simulink model "VirtualADAPT"  (Simulink 8.9)
settings: Solver=ode45  StartTime=0.0  StopTime=inf  RelTol=1e-3  EnableMultiTasking=on
PostLoadFcn:
  | FaultInjectionGUIHandle = FaultInjectionGUI;
InitFcn:
  | VirtualADAPTDataStructure
undefined symbols (not defined in the file or in its folder): ln
libraries: simulink x363
(part 8 of 26)

== system VirtualADAPT/VirtualADAPTv1/PowerStorage/RelayEY260/Relay ==
  u(1) : Inport
  u(2) : Inport  Port=2
  u(3) : Inport  Port=3
  u(4) : Inport  Port=4
  Adder : Sum  SaturateOnIntegerOverflow=on  InputSameDT=on  OutDataTypeStr=Inherit: Same as first input
  Gain : Gain  SaturateOnIntegerOverflow=on  OutDataTypeStr=Inherit: Same as input  ParamDataTypeStr=Inherit: Same as input
  Mux : Mux
  OffGuard : Fcn  Expr=(!u(4))
  OnGuard : Fcn  Expr=(u(4))
  Product1 : Product  SaturateOnIntegerOverflow=on  RndMeth=Zero  InputSameDT=on  OutDataTypeStr=Inherit: Same as first input
  Product2 : Product  SaturateOnIntegerOverflow=on  RndMeth=Zero  InputSameDT=on  OutDataTypeStr=Inherit: Same as first input
  determineState : Subsystem
  y(1) : Outport
  y(2) : Outport  Port=2
  y(3) : Outport  Port=3
  O : Outport  Port=4
  State : Outport  Port=5
  wires:
  Adder/1 -> Gain/1
  u(4)/1 -> Mux/4
  Mux/1 -> OnGuard/1, OffGuard/1
  OnGuard/1 -> determineState/1(In1)
  OffGuard/1 -> determineState/2(In2)
  u(1)/1 -> Product1/1
  determineState/1(Out1) -> Product1/2, Product2/2, State/1
  Product1/1 -> O/1, y(2)/1, y(3)/1
  Gain/1 -> Product2/1
  Product2/1 -> y(1)/1
  u(2)/1 -> Adder/1
  u(3)/1 -> Adder/2

== system VirtualADAPT/VirtualADAPTv1/PowerStorage/RelayEY260/Sensor ==
  TrueValue : Inport
  MSe : Subsystem  mask{FP=Nominal, FT=0, FM=0}
      mask prompts: FP "Fault Profile:"; FT "Fault Injection Time:"; FM "Fault Magnitude/Slope/Bias/StuckAtValue"
      mask TypeOptions FP: Nominal | Abrupt | Incipient | Bias | StuckAt
      mask Display: image(imread('MSe.png','BackgroundColor',[1 1 1]))
  R : Subsystem  mask{R=1, FP=Nominal, FT=0, FM=0}
      mask prompts: R "Value of R:"; FP "Fault Profile:"; FT "Fault Injection Time:"; FM "Fault Magnitude/Slope"
      mask TypeOptions FP: Nominal | Abrupt | Incipient
      mask Display: image(imread('R.png','BackgroundColor',[1 1 1]))
  TrueValueMod : Subsystem
      mask Display: image(imread('fx.png','BackgroundColor',[1 1 1]))
  Value : Subsystem
      mask Display: image(imread('0.png','BackgroundColor',[1 1 1]))
  SensedValue : Outport
  wires:
  Value/1(y(1)) -> R/1(In)
  R/1(Out) -> Value/1(u(1))
  MSe/1(E) -> Value/2(u(2))
  Value/2(y(2)) -> MSe/1(F)
  TrueValueMod/1(y(1)) -> MSe/2(Se)
  TrueValue/1 -> TrueValueMod/1(u(1))
  Value/3(O) -> SensedValue/1

== system VirtualADAPT/VirtualADAPTv1/PowerStorage/SensorE161/MSe ==
  F : Inport
  Se : Inport  Port=2
  Bias : Step  Time=FT  After=FM  SampleTime=0
  Fault Inject : Subsystem
  Fault Profile : Constant  Value=FP
  Mult2 : Product  SaturateOnIntegerOverflow=on  RndMeth=Zero  InputSameDT=on  OutDataTypeStr=Inherit: Same as first input
  Multiply : Product  SaturateOnIntegerOverflow=on  RndMeth=Zero  InputSameDT=on  OutDataTypeStr=Inherit: Same as first input
  Ramp : simulink/Sources/Ramp  slope=FM  start=FT  X0=0
  Step : Step  Time=FT  Before=1  After=(1 + FM)  SampleTime=0
  StuckAt : Step  Time=FT  Before=1  After=0  SampleTime=0
  StuckAt2 : Step  Time=FT  After=FM  SampleTime=0
  Sum : Sum  SaturateOnIntegerOverflow=on  InputSameDT=on  OutDataTypeStr=Inherit: Same as first input
  Sum2 : Sum  SaturateOnIntegerOverflow=on  InputSameDT=on  OutDataTypeStr=Inherit: Same as first input
  Sum3 : Sum  SaturateOnIntegerOverflow=on  InputSameDT=on  OutDataTypeStr=Inherit: Same as first input
  E : Outport
  wires:
  Fault Inject/1(Out1) -> E/1
  Se/1 -> Fault Inject/1(Nominal), Multiply/1, Sum/1, Sum2/1, Mult2/1
  Fault Profile/1 -> Fault Inject/2(Fault Profile)
  Step/1 -> Multiply/2
  Ramp/1 -> Sum/2
  Multiply/1 -> Fault Inject/3(Step)
  Sum/1 -> Fault Inject/4(Ramp)
  Bias/1 -> Sum2/2
  Sum2/1 -> Fault Inject/5(Bias)
  StuckAt/1 -> Mult2/2
  Mult2/1 -> Sum3/1
  StuckAt2/1 -> Sum3/2
  Sum3/1 -> Fault Inject/6(stuckAt)

== system VirtualADAPT/VirtualADAPTv1/PowerStorage/SensorE161/R ==
  In : Inport
  Bond : Constant  Value=2
  Computing R : Subsystem
  Fault Profile : Constant  Value=FP
  JuncDB : Constant
  Junction Type : Constant  Value=0
  Ramp : simulink/Sources/Ramp  slope=FM  start=FT  X0=R
  Resistor Value : Constant  Value=R
  Step : Step  Time=FT  Before=R  After=(1 + FM)*R  SampleTime=0
  Out : Outport
  R : Outport  Port=2
  wires:
  Computing R/1(Out1) -> Out/1
  In/1 -> Computing R/1(In1)
  Resistor Value/1 -> Computing R/2(RNominal)
  Fault Profile/1 -> Computing R/3(Fault Profile)
  Step/1 -> Computing R/4(Step)
  Ramp/1 -> Computing R/5(Ramp)
  JuncDB/1 -> Computing R/6(JuncDB)
  Bond/1 -> Computing R/7(Bond)
  Junction Type/1 -> Computing R/8(Junction Type)
  Computing R/2(Out2) -> R/1

== system VirtualADAPT/VirtualADAPTv1/PowerStorage/SensorE161/TrueValueMod ==
  u(1) : Inport
  Function : Fcn  Expr=u(1)
  Mux : Mux  Inputs=1
  y(1) : Outport
  wires:
  u(1)/1 -> Mux/1
  Mux/1 -> Function/1
  Function/1 -> y(1)/1

== system VirtualADAPT/VirtualADAPTv1/PowerStorage/SensorE161/Value ==
  u(1) : Inport
  u(2) : Inport  Port=2
  Adder : Sum  Inputs=-  SaturateOnIntegerOverflow=on  InputSameDT=on  OutDataTypeStr=Inherit: Same as first input
  Gain : Gain  Gain=-1  SaturateOnIntegerOverflow=on  OutDataTypeStr=Inherit: Same as input  ParamDataTypeStr=Inherit: Same as input
  y(1) : Outport
  y(2) : Outport  Port=2
  O : Outport  Port=3
  wires:
  Adder/1 -> Gain/1
  Gain/1 -> y(1)/1
  u(1)/1 -> y(2)/1, O/1
  u(2)/1 -> Adder/1

== system VirtualADAPT/VirtualADAPTv1/PowerStorage/SensorE261/MSe ==
  F : Inport
  Se : Inport  Port=2
  Bias : Step  Time=FT  After=FM  SampleTime=0
  Fault Inject : Subsystem
  Fault Profile : Constant  Value=FP
  Mult2 : Product  SaturateOnIntegerOverflow=on  RndMeth=Zero  InputSameDT=on  OutDataTypeStr=Inherit: Same as first input
  Multiply : Product  SaturateOnIntegerOverflow=on  RndMeth=Zero  InputSameDT=on  OutDataTypeStr=Inherit: Same as first input
  Ramp : simulink/Sources/Ramp  slope=FM  start=FT  X0=0
  Step : Step  Time=FT  Before=1  After=(1 + FM)  SampleTime=0
  StuckAt : Step  Time=FT  Before=1  After=0  SampleTime=0
  StuckAt2 : Step  Time=FT  After=FM  SampleTime=0
  Sum : Sum  SaturateOnIntegerOverflow=on  InputSameDT=on  OutDataTypeStr=Inherit: Same as first input
  Sum2 : Sum  SaturateOnIntegerOverflow=on  InputSameDT=on  OutDataTypeStr=Inherit: Same as first input
  Sum3 : Sum  SaturateOnIntegerOverflow=on  InputSameDT=on  OutDataTypeStr=Inherit: Same as first input
  E : Outport
  wires:
  Fault Inject/1(Out1) -> E/1
  Se/1 -> Fault Inject/1(Nominal), Multiply/1, Sum/1, Sum2/1, Mult2/1
  Fault Profile/1 -> Fault Inject/2(Fault Profile)
  Step/1 -> Multiply/2
  Ramp/1 -> Sum/2
  Multiply/1 -> Fault Inject/3(Step)
  Sum/1 -> Fault Inject/4(Ramp)
  Bias/1 -> Sum2/2
  Sum2/1 -> Fault Inject/5(Bias)
  StuckAt/1 -> Mult2/2
  Mult2/1 -> Sum3/1
  StuckAt2/1 -> Sum3/2
  Sum3/1 -> Fault Inject/6(stuckAt)

== system VirtualADAPT/VirtualADAPTv1/PowerStorage/SensorE261/R ==
  In : Inport
  Bond : Constant  Value=2
  Computing R : Subsystem
  Fault Profile : Constant  Value=FP
  JuncDB : Constant
  Junction Type : Constant  Value=0
  Ramp : simulink/Sources/Ramp  slope=FM  start=FT  X0=R
  Resistor Value : Constant  Value=R
  Step : Step  Time=FT  Before=R  After=(1 + FM)*R  SampleTime=0
  Out : Outport
  R : Outport  Port=2
  wires:
  Computing R/1(Out1) -> Out/1
  In/1 -> Computing R/1(In1)
  Resistor Value/1 -> Computing R/2(RNominal)
  Fault Profile/1 -> Computing R/3(Fault Profile)
  Step/1 -> Computing R/4(Step)
  Ramp/1 -> Computing R/5(Ramp)
  JuncDB/1 -> Computing R/6(JuncDB)
  Bond/1 -> Computing R/7(Bond)
  Junction Type/1 -> Computing R/8(Junction Type)
  Computing R/2(Out2) -> R/1

== system VirtualADAPT/VirtualADAPTv1/PowerStorage/SensorE261/TrueValueMod ==
  u(1) : Inport
  Function : Fcn  Expr=u(1)
  Mux : Mux  Inputs=1
  y(1) : Outport
  wires:
  u(1)/1 -> Mux/1
  Mux/1 -> Function/1
  Function/1 -> y(1)/1

== system VirtualADAPT/VirtualADAPTv1/PowerStorage/SensorE261/Value ==
  u(1) : Inport
  u(2) : Inport  Port=2
  Adder : Sum  Inputs=-  SaturateOnIntegerOverflow=on  InputSameDT=on  OutDataTypeStr=Inherit: Same as first input
  Gain : Gain  Gain=-1  SaturateOnIntegerOverflow=on  OutDataTypeStr=Inherit: Same as input  ParamDataTypeStr=Inherit: Same as input
  y(1) : Outport
  y(2) : Outport  Port=2
  O : Outport  Port=3
  wires:
  Adder/1 -> Gain/1
  Gain/1 -> y(1)/1
  u(1)/1 -> y(2)/1, O/1
  u(2)/1 -> Adder/1

== system VirtualADAPT/VirtualADAPTv1/PowerStorage/SensorIT161/MSe ==
  F : Inport
  Se : Inport  Port=2
  Bias : Step  Time=FT  After=FM  SampleTime=0
  Fault Inject : Subsystem
  Fault Profile : Constant  Value=FP
  Mult2 : Product  SaturateOnIntegerOverflow=on  RndMeth=Zero  InputSameDT=on  OutDataTypeStr=Inherit: Same as first input
  Multiply : Product  SaturateOnIntegerOverflow=on  RndMeth=Zero  InputSameDT=on  OutDataTypeStr=Inherit: Same as first input
  Ramp : simulink/Sources/Ramp  slope=FM  start=FT  X0=0
  Step : Step  Time=FT  Before=1  After=(1 + FM)  SampleTime=0
  StuckAt : Step  Time=FT  Before=1  After=0  SampleTime=0
  StuckAt2 : Step  Time=FT  After=FM  SampleTime=0
  Sum : Sum  SaturateOnIntegerOverflow=on  InputSameDT=on  OutDataTypeStr=Inherit: Same as first input
  Sum2 : Sum  SaturateOnIntegerOverflow=on  InputSameDT=on  OutDataTypeStr=Inherit: Same as first input
  Sum3 : Sum  SaturateOnIntegerOverflow=on  InputSameDT=on  OutDataTypeStr=Inherit: Same as first input
  E : Outport
  wires:
  Fault Inject/1(Out1) -> E/1
  Se/1 -> Fault Inject/1(Nominal), Multiply/1, Sum/1, Sum2/1, Mult2/1
  Fault Profile/1 -> Fault Inject/2(Fault Profile)
  Step/1 -> Multiply/2
  Ramp/1 -> Sum/2
  Multiply/1 -> Fault Inject/3(Step)
  Sum/1 -> Fault Inject/4(Ramp)
  Bias/1 -> Sum2/2
  Sum2/1 -> Fault Inject/5(Bias)
  StuckAt/1 -> Mult2/2
  Mult2/1 -> Sum3/1
  StuckAt2/1 -> Sum3/2
  Sum3/1 -> Fault Inject/6(stuckAt)

== system VirtualADAPT/VirtualADAPTv1/PowerStorage/SensorIT161/R ==
  In : Inport
  Bond : Constant  Value=2
  Computing R : Subsystem
  Fault Profile : Constant  Value=FP
  JuncDB : Constant
  Junction Type : Constant  Value=0
  Ramp : simulink/Sources/Ramp  slope=FM  start=FT  X0=R
  Resistor Value : Constant  Value=R
  Step : Step  Time=FT  Before=R  After=(1 + FM)*R  SampleTime=0
  Out : Outport
  R : Outport  Port=2
  wires:
  Computing R/1(Out1) -> Out/1
  In/1 -> Computing R/1(In1)
  Resistor Value/1 -> Computing R/2(RNominal)
  Fault Profile/1 -> Computing R/3(Fault Profile)
  Step/1 -> Computing R/4(Step)
  Ramp/1 -> Computing R/5(Ramp)
  JuncDB/1 -> Computing R/6(JuncDB)
  Bond/1 -> Computing R/7(Bond)
  Junction Type/1 -> Computing R/8(Junction Type)
  Computing R/2(Out2) -> R/1

== system VirtualADAPT/VirtualADAPTv1/PowerStorage/SensorIT161/TrueValueMod ==
  u(1) : Inport
  Function : Fcn  Expr=u(1)
  Mux : Mux  Inputs=1
  y(1) : Outport
  wires:
  u(1)/1 -> Mux/1
  Mux/1 -> Function/1
  Function/1 -> y(1)/1

== system VirtualADAPT/VirtualADAPTv1/PowerStorage/SensorIT161/Value ==
  u(1) : Inport
  u(2) : Inport  Port=2
  Adder : Sum  Inputs=-  SaturateOnIntegerOverflow=on  InputSameDT=on  OutDataTypeStr=Inherit: Same as first input
  Gain : Gain  Gain=-1  SaturateOnIntegerOverflow=on  OutDataTypeStr=Inherit: Same as input  ParamDataTypeStr=Inherit: Same as input
  y(1) : Outport
  y(2) : Outport  Port=2
  O : Outport  Port=3
  wires:
  Adder/1 -> Gain/1
  Gain/1 -> y(1)/1
  u(1)/1 -> y(2)/1, O/1
  u(2)/1 -> Adder/1

== system VirtualADAPT/VirtualADAPTv1/PowerStorage/SensorIT261/MSe ==
  F : Inport
  Se : Inport  Port=2
  Bias : Step  Time=FT  After=FM  SampleTime=0
  Fault Inject : Subsystem
  Fault Profile : Constant  Value=FP
  Mult2 : Product  SaturateOnIntegerOverflow=on  RndMeth=Zero  InputSameDT=on  OutDataTypeStr=Inherit: Same as first input
  Multiply : Product  SaturateOnIntegerOverflow=on  RndMeth=Zero  InputSameDT=on  OutDataTypeStr=Inherit: Same as first input
  Ramp : simulink/Sources/Ramp  slope=FM  start=FT  X0=0
  Step : Step  Time=FT  Before=1  After=(1 + FM)  SampleTime=0
  StuckAt : Step  Time=FT  Before=1  After=0  SampleTime=0
  StuckAt2 : Step  Time=FT  After=FM  SampleTime=0
  Sum : Sum  SaturateOnIntegerOverflow=on  InputSameDT=on  OutDataTypeStr=Inherit: Same as first input
  Sum2 : Sum  SaturateOnIntegerOverflow=on  InputSameDT=on  OutDataTypeStr=Inherit: Same as first input
  Sum3 : Sum  SaturateOnIntegerOverflow=on  InputSameDT=on  OutDataTypeStr=Inherit: Same as first input
  E : Outport
  wires:
  Fault Inject/1(Out1) -> E/1
  Se/1 -> Fault Inject/1(Nominal), Multiply/1, Sum/1, Sum2/1, Mult2/1
  Fault Profile/1 -> Fault Inject/2(Fault Profile)
  Step/1 -> Multiply/2
  Ramp/1 -> Sum/2
  Multiply/1 -> Fault Inject/3(Step)
  Sum/1 -> Fault Inject/4(Ramp)
  Bias/1 -> Sum2/2
  Sum2/1 -> Fault Inject/5(Bias)
  StuckAt/1 -> Mult2/2
  Mult2/1 -> Sum3/1
  StuckAt2/1 -> Sum3/2
  Sum3/1 -> Fault Inject/6(stuckAt)

== system VirtualADAPT/VirtualADAPTv1/PowerStorage/SensorIT261/R ==
  In : Inport
  Bond : Constant  Value=2
  Computing R : Subsystem
  Fault Profile : Constant  Value=FP
  JuncDB : Constant
  Junction Type : Constant  Value=0
  Ramp : simulink/Sources/Ramp  slope=FM  start=FT  X0=R
  Resistor Value : Constant  Value=R
  Step : Step  Time=FT  Before=R  After=(1 + FM)*R  SampleTime=0
  Out : Outport
  R : Outport  Port=2
  wires:
  Computing R/1(Out1) -> Out/1
  In/1 -> Computing R/1(In1)
  Resistor Value/1 -> Computing R/2(RNominal)
  Fault Profile/1 -> Computing R/3(Fault Profile)
  Step/1 -> Computing R/4(Step)
  Ramp/1 -> Computing R/5(Ramp)
  JuncDB/1 -> Computing R/6(JuncDB)
  Bond/1 -> Computing R/7(Bond)
  Junction Type/1 -> Computing R/8(Junction Type)
  Computing R/2(Out2) -> R/1

== system VirtualADAPT/VirtualADAPTv1/PowerStorage/SensorIT261/TrueValueMod ==
  u(1) : Inport
  Function : Fcn  Expr=u(1)
  Mux : Mux  Inputs=1
  y(1) : Outport
  wires:
  u(1)/1 -> Mux/1
  Mux/1 -> Function/1
  Function/1 -> y(1)/1

== system VirtualADAPT/VirtualADAPTv1/PowerStorage/SensorIT261/Value ==
  u(1) : Inport
  u(2) : Inport  Port=2
  Adder : Sum  Inputs=-  SaturateOnIntegerOverflow=on  InputSameDT=on  OutDataTypeStr=Inherit: Same as first input
  Gain : Gain  Gain=-1  SaturateOnIntegerOverflow=on  OutDataTypeStr=Inherit: Same as input  ParamDataTypeStr=Inherit: Same as input
  y(1) : Outport
  y(2) : Outport  Port=2
  O : Outport  Port=3
  wires:
  Adder/1 -> Gain/1
  Gain/1 -> y(1)/1
  u(1)/1 -> y(2)/1, O/1
  u(2)/1 -> Adder/1

== system VirtualADAPT/VirtualADAPTv1/SensorTE133/MSe/Fault Inject ==
  Nominal : Inport
  Fault Profile : Inport  Port=2
  Step : Inport  Port=3
  Ramp : Inport  Port=4
  Bias : Inport  Port=5
  stuckAt : Inport  Port=6
  Switch1 : MultiPortSwitch  Inputs=5  SaturateOnIntegerOverflow=on  InputSameDT=on
  Out1 : Outport
  wires:
  Nominal/1 -> Switch1/2
  Fault Profile/1 -> Switch1/1
  Step/1 -> Switch1/3
  Ramp/1 -> Switch1/4
  Bias/1 -> Switch1/5
  stuckAt/1 -> Switch1/6
  Switch1/1 -> Out1/1

== system VirtualADAPT/VirtualADAPTv1/SensorTE133/R/Computing R ==
  In1 : Inport
  RNominal : Inport  Port=2
  Fault Profile : Inport  Port=3
  Step : Inport  Port=4
  Ramp : Inport  Port=5
  JuncDB : Inport  Port=6
  Bond : Inport  Port=7
  Junction Type : Inport  Port=8
  CalcCausality : Fcn  Expr=(u(1) == u(2) && u(3) ==1) || (u(1) !=u(2) && u(3) == 0)
  CalcOutput : Fcn  Expr=(u(1)*u(2)^u(3))
  MinusOne : Constant  Value=-1
  Mux1 : Mux  Inputs=3
  Mux2 : Mux  Inputs=3
  One : Constant
  Switch1 : MultiPortSwitch  SaturateOnIntegerOverflow=on  InputSameDT=on
  Switch2 : MultiPortSwitch  DataPortOrder=Zero-based contiguous  Inputs=2  zeroidx=on  SaturateOnIntegerOverflow=on  InputSameDT=on
  Out1 : Outport
  Out2 : Outport  Port=2
  wires:
  In1/1 -> Mux2/1
  Fault Profile/1 -> Switch1/1
  RNominal/1 -> Switch1/2
  Step/1 -> Switch1/3
  Ramp/1 -> Switch1/4
  Switch1/1 -> Out2/1, Mux2/2
  JuncDB/1 -> Mux1/1
  Bond/1 -> Mux1/2
  Junction Type/1 -> Mux1/3
  Mux1/1 -> CalcCausality/1
  CalcCausality/1 -> Switch2/1
  One/1 -> Switch2/2
  MinusOne/1 -> Switch2/3
  Switch2/1 -> Mux2/3
  Mux2/1 -> CalcOutput/1
  CalcOutput/1 -> Out1/1

== system VirtualADAPT/VirtualADAPTv1/SensorTE133/TempBias/Fault Inject ==
  Nominal : Inport
  Fault Profile : Inport  Port=2
  Step : Inport  Port=3
  Ramp : Inport  Port=4
  Bias : Inport  Port=5
  stuckAt : Inport  Port=6
  Switch1 : MultiPortSwitch  Inputs=5  SaturateOnIntegerOverflow=on  InputSameDT=on
  Out1 : Outport
  wires:
  Nominal/1 -> Switch1/2
  Fault Profile/1 -> Switch1/1
  Step/1 -> Switch1/3
  Ramp/1 -> Switch1/4
  Bias/1 -> Switch1/5
  stuckAt/1 -> Switch1/6
  Switch1/1 -> Out1/1

== system VirtualADAPT/VirtualADAPTv1/PowerDistribution/ACLoadBank1/L1A/LGT6 ==
  VInIn0 : Inport
  AmbientTemp : Inport  Port=2
  ComputeCurrent : Subsystem
      mask Display: image(imread('fx.png','BackgroundColor',[1 1 1]))
  ComputePower : Subsystem
      mask Display: image(imread('fx.png','BackgroundColor',[1 1 1]))
  LightBulb1 : Subsystem
  LightBulb2 : Subsystem
  LightBulb3 : Subsystem
  O1 : Subsystem
      mask Display: image(imread('1.png','BackgroundColor',[1 1 1]))
  Z1 : Subsystem
      mask Display: image(imread('0.png','BackgroundColor',[1 1 1]))
  VInOut0 : Outport
  Power : Outport  Port=2
  Temperature1 : Outport  Port=3
  Temperature3 : Outport  Port=4
  Current : Outport  Port=5
  Temperature2 : Outport  Port=6
  wires:
  O1/1(y(1)) -> Z1/1(u(1))
  Z1/1(y(1)) -> O1/1(u(1))
  VInIn0/1 -> O1/2(u(2))
  O1/2(y(2)) -> VInOut0/1
  LightBulb1/1(EPOut0) -> Z1/2(u(2))
  Z1/2(y(2)) -> LightBulb1/1(EPIn0)
  LightBulb2/1(EPOut0) -> Z1/3(u(3))
  Z1/3(y(3)) -> LightBulb2/1(EPIn0)
  LightBulb3/1(EPOut0) -> Z1/4(u(4))
  Z1/4(y(4)) -> LightBulb3/1(EPIn0)
  LightBulb1/4(Power) -> ComputePower/1(u(1))
  LightBulb2/4(Power) -> ComputePower/2(u(2))
  LightBulb3/2(Power) -> ComputePower/3(u(3))
  LightBulb1/2(Current) -> ComputeCurrent/1(u(1))
  LightBulb2/3(Current) -> ComputeCurrent/2(u(2))
  LightBulb3/3(Current) -> ComputeCurrent/3(u(3))
  ComputePower/1(y(1)) -> Power/1
  LightBulb1/3(Temperature) -> Temperature1/1
  LightBulb2/2(Temperature) -> Temperature2/1
  LightBulb3/4(Temperature) -> Temperature3/1
  AmbientTemp/1 -> LightBulb1/2(AmbientTemp), LightBulb2/2(AmbientTemp), LightBulb3/2(AmbientTemp)
  ComputeCurrent/1(y(1)) -> Current/1

== system VirtualADAPT/VirtualADAPTv1/PowerDistribution/ACLoadBank1/L1A/SensorLT500 ==
  Power : Inport
  Bias : Subsystem  mask{Constant=15.411, FP=Nominal, FT=0, FM=0}
      mask prompts: Constant "Value of Constant:"; FP "Fault Profile:"; FT "Fault Injection Time:"; FM "Fault Magnitude/Slope"
      mask TypeOptions FP: Nominal | Abrupt | Incipient
      mask Display: image(imread('Constant.png','BackgroundColor',[1 1 1]))
  ComputeLuminosity : Subsystem
      mask Display: image(imread('fx.png','BackgroundColor',[1 1 1]))
  Gain : Subsystem  mask{Constant=0.56045, FP=Nominal, FT=0, FM=0}
      mask prompts: Constant "Value of Constant:"; FP "Fault Profile:"; FT "Fault Injection Time:"; FM "Fault Magnitude/Slope"
      mask TypeOptions FP: Nominal | Abrupt | Incipient
      mask Display: image(imread('Constant.png','BackgroundColor',[1 1 1]))
  MSe : Subsystem  mask{FP=Nominal, FT=0, FM=0}
      mask prompts: FP "Fault Profile:"; FT "Fault Injection Time:"; FM "Fault Magnitude/Slope/Bias/StuckAtValue"
      mask TypeOptions FP: Nominal | Abrupt | Incipient | Bias | StuckAt
      mask Display: image(imread('MSe.png','BackgroundColor',[1 1 1]))
  R : Subsystem  mask{R=100, FP=Nominal, FT=0, FM=0}
      mask prompts: R "Value of R:"; FP "Fault Profile:"; FT "Fault Injection Time:"; FM "Fault Magnitude/Slope"
      mask TypeOptions FP: Nominal | Abrupt | Incipient
      mask Display: image(imread('R.png','BackgroundColor',[1 1 1]))
  ZeroJunction : Subsystem
      mask Display: image(imread('0.png','BackgroundColor',[1 1 1]))
  Light : Outport
  wires:
  ZeroJunction/1(y(1)) -> R/1(In)
  R/1(Out) -> ZeroJunction/1(u(1))
  MSe/1(E) -> ZeroJunction/2(u(2))
  ZeroJunction/2(y(2)) -> MSe/1(F)
  ComputeLuminosity/1(y(1)) -> MSe/2(Se)
  Bias/1(C) -> ComputeLuminosity/1(u(1))
  Power/1 -> ComputeLuminosity/2(u(2))
  Gain/1(C) -> ComputeLuminosity/3(u(3))
  ZeroJunction/3(O) -> Light/1

== system VirtualADAPT/VirtualADAPTv1/PowerDistribution/ACLoadBank1/L1A/SensorTE500 ==
  TrueValue : Inport
  MSe : Subsystem  mask{FP=Nominal, FT=0, FM=0}
      mask prompts: FP "Fault Profile:"; FT "Fault Injection Time:"; FM "Fault Magnitude/Slope/Bias/StuckAtValue"
      mask TypeOptions FP: Nominal | Abrupt | Incipient | Bias | StuckAt
      mask Display: image(imread('MSe.png','BackgroundColor',[1 1 1]))
  R : Subsystem  mask{R=1, FP=Nominal, FT=0, FM=0}
      mask prompts: R "Value of R:"; FP "Fault Profile:"; FT "Fault Injection Time:"; FM "Fault Magnitude/Slope"
      mask TypeOptions FP: Nominal | Abrupt | Incipient
      mask Display: image(imread('R.png','BackgroundColor',[1 1 1]))
  TempBias : Subsystem  mask{Constant=-1.5, FP=Nominal, FT=0, FM=0}
      mask prompts: Constant "Value of Constant:"; FP "Fault Profile:"; FT "Fault Injection Time:"; FM "Fault Magnitude/Slope"
      mask TypeOptions FP: Nominal | Abrupt | Incipient
      mask Display: image(imread('Constant.png','BackgroundColor',[1 1 1]))
  TrueValueMod : Subsystem
      mask Display: image(imread('fx.png','BackgroundColor',[1 1 1]))
  Value : Subsystem
      mask Display: image(imread('0.png','BackgroundColor',[1 1 1]))
  SensedValue : Outport
  wires:
  MSe/1(E) -> Value/1(u(1))
  Value/1(y(1)) -> MSe/1(F)
  Value/2(y(2)) -> R/1(In)
  R/1(Out) -> Value/2(u(2))
  TrueValueMod/1(y(1)) -> MSe/2(Se)
  TempBias/1(C) -> TrueValueMod/1(u(1))
  TrueValue/1 -> TrueValueMod/2(u(2))
  Value/3(O) -> SensedValue/1

== system VirtualADAPT/VirtualADAPTv1/PowerDistribution/ACLoadBank1/L1A/SensorTE501 ==
  TrueValue : Inport
  MSe : Subsystem  mask{FP=Nominal, FT=0, FM=0}
      mask prompts: FP "Fault Profile:"; FT "Fault Injection Time:"; FM "Fault Magnitude/Slope/Bias/StuckAtValue"
      mask TypeOptions FP: Nominal | Abrupt | Incipient | Bias | StuckAt
      mask Display: image(imread('MSe.png','BackgroundColor',[1 1 1]))
  R : Subsystem  mask{R=1, FP=Nominal, FT=0, FM=0}
      mask prompts: R "Value of R:"; FP "Fault Profile:"; FT "Fault Injection Time:"; FM "Fault Magnitude/Slope"
      mask TypeOptions FP: Nominal | Abrupt | Incipient
      mask Display: image(imread('R.png','BackgroundColor',[1 1 1]))
  TempBias : Subsystem  mask{Constant=-1.33, FP=Nominal, FT=0, FM=0}
      mask prompts: Constant "Value of Constant:"; FP "Fault Profile:"; FT "Fault Injection Time:"; FM "Fault Magnitude/Slope"
      mask TypeOptions FP: Nominal | Abrupt | Incipient
      mask Display: image(imread('Constant.png','BackgroundColor',[1 1 1]))
  TrueValueMod : Subsystem
      mask Display: image(imread('fx.png','BackgroundColor',[1 1 1]))
  Value : Subsystem
      mask Display: image(imread('0.png','BackgroundColor',[1 1 1]))
  SensedValue : Outport
  wires:
  MSe/1(E) -> Value/1(u(1))
  Value/1(y(1)) -> MSe/1(F)
  Value/2(y(2)) -> R/1(In)
  R/1(Out) -> Value/2(u(2))
  TrueValueMod/1(y(1)) -> MSe/2(Se)
  TempBias/1(C) -> TrueValueMod/1(u(1))
  TrueValue/1 -> TrueValueMod/2(u(2))
  Value/3(O) -> SensedValue/1

== system VirtualADAPT/VirtualADAPTv1/PowerDistribution/ACLoadBank1/L1A/SensorTE502 ==
  TrueValue : Inport
  MSe : Subsystem  mask{FP=Nominal, FT=0, FM=0}
      mask prompts: FP "Fault Profile:"; FT "Fault Injection Time:"; FM "Fault Magnitude/Slope/Bias/StuckAtValue"
      mask TypeOptions FP: Nominal | Abrupt | Incipient | Bias | StuckAt
      mask Display: image(imread('MSe.png','BackgroundColor',[1 1 1]))
  R : Subsystem  mask{R=1, FP=Nominal, FT=0, FM=0}
      mask prompts: R "Value of R:"; FP "Fault Profile:"; FT "Fault Injection Time:"; FM "Fault Magnitude/Slope"
      mask TypeOptions FP: Nominal | Abrupt | Incipient
      mask Display: image(imread('R.png','BackgroundColor',[1 1 1]))
  TempBias : Subsystem  mask{Constant=-2.05, FP=Nominal, FT=0, FM=0}
      mask prompts: Constant "Value of Constant:"; FP "Fault Profile:"; FT "Fault Injection Time:"; FM "Fault Magnitude/Slope"
      mask TypeOptions FP: Nominal | Abrupt | Incipient
      mask Display: image(imread('Constant.png','BackgroundColor',[1 1 1]))
  TrueValueMod : Subsystem
      mask Display: image(imread('fx.png','BackgroundColor',[1 1 1]))
  Value : Subsystem
      mask Display: image(imread('0.png','BackgroundColor',[1 1 1]))
  SensedValue : Outport
  wires:
  MSe/1(E) -> Value/1(u(1))
  Value/1(y(1)) -> MSe/1(F)
  Value/2(y(2)) -> R/1(In)
  R/1(Out) -> Value/2(u(2))
  TrueValueMod/1(y(1)) -> MSe/2(Se)
  TempBias/1(C) -> TrueValueMod/1(u(1))
  TrueValue/1 -> TrueValueMod/2(u(2))
  Value/3(O) -> SensedValue/1

== system VirtualADAPT/VirtualADAPTv1/PowerDistribution/ACLoadBank1/L1B/FAN1 ==
  InputIn0 : Inport
  FanRotationalVelocity : Subsystem
      mask Display: image(imread('1.png','BackgroundColor',[1 1 1]))
  Friction : Subsystem  mask{R=0.00016811, FP=Nominal, FT=0, FM=0}
      mask prompts: R "Value of R:"; FP "Fault Profile:"; FT "Fault Injection Time:"; FM "Fault Magnitude/Slope"
      mask TypeOptions FP: Nominal | Abrupt | Incipient
      mask Display: image(imread('R.png','BackgroundColor',[1 1 1]))
  GY : Subsystem  mask{GY=0.0307, FP=Nominal, FT=0, FM=0}
      mask prompts: GY "Value of GY:"; FP "Fault Profile:"; FT "Fault Injection Time:"; FM "Fault Magnitude/Slope"
      mask TypeOptions FP: Nominal | Abrupt | Incipient
      mask Display: image(imread('GY.png','BackgroundColor',[1 1 1]))
  Inductance : Subsystem  mask{I=18.228, IC=0, FP=Nominal, FT=0, FM=0}
      mask prompts: I "Value of I:"; IC "Initial Condition:"; FP "Fault Profile:"; FT "Fault Injection Time:"; FM "Fault Magnitude/Slope"
      mask TypeOptions FP: Nominal | Abrupt | Incipient
      mask Display: image(imread('I.png','BackgroundColor',[1 1 1]))
  Inertia : Subsystem  mask{I=0.00047618, IC=0, FP=Nominal, FT=0, FM=0}
      mask prompts: I "Value of I:"; IC "Initial Condition:"; FP "Fault Profile:"; FT "Fault Injection Time:"; FM "Fault Magnitude/Slope"
      mask TypeOptions FP: Nominal | Abrupt | Incipient
      mask Display: image(imread('I.png','BackgroundColor',[1 1 1]))
  MotorCurrent : Subsystem
      mask Display: image(imread('1.png','BackgroundColor',[1 1 1]))
  Resistance : Subsystem  mask{R=195, FP=Nominal, FT=0, FM=0}
      mask prompts: R "Value of R:"; FP "Fault Profile:"; FT "Fault Injection Time:"; FM "Fault Magnitude/Slope"
      mask TypeOptions FP: Nominal | Abrupt | Incipient
      mask Display: image(imread('R.png','BackgroundColor',[1 1 1]))
  InputOut0 : Outport
  RotationalVelocity : Outport  Port=2
  Current : Outport  Port=3
  wires:
  MotorCurrent/1(y(1)) -> Inductance/1(E)
  Inductance/1(F) -> MotorCurrent/1(u(1))
  FanRotationalVelocity/1(y(1)) -> Inertia/1(E)
  Inertia/1(F) -> FanRotationalVelocity/1(u(1))
  MotorCurrent/2(y(2)) -> GY/1(I1)
  GY/1(O1) -> MotorCurrent/2(u(2))
  GY/2(O2) -> FanRotationalVelocity/2(u(2))
  FanRotationalVelocity/2(y(2)) -> GY/2(I2)
  MotorCurrent/3(y(3)) -> Resistance/1(In)
  Resistance/1(Out) -> MotorCurrent/3(u(3))
  FanRotationalVelocity/3(y(3)) -> Friction/1(In)
  Friction/1(Out) -> FanRotationalVelocity/3(u(3))
  InputIn0/1 -> MotorCurrent/4(u(4))
  MotorCurrent/4(y(4)) -> InputOut0/1
  FanRotationalVelocity/4(O) -> RotationalVelocity/1
  MotorCurrent/5(O) -> Current/1

== system VirtualADAPT/VirtualADAPTv1/PowerDistribution/ACLoadBank1/L1B/SensorST515 ==
  TrueValue : Inport
  Bias : Subsystem  mask{Constant=0.60132, FP=Nominal, FT=0, FM=0}
      mask prompts: Constant "Value of Constant:"; FP "Fault Profile:"; FT "Fault Injection Time:"; FM "Fault Magnitude/Slope"
      mask TypeOptions FP: Nominal | Abrupt | Incipient
      mask Display: image(imread('Constant.png','BackgroundColor',[1 1 1]))
  Gain : Subsystem  mask{Constant=3.7986, FP=Nominal, FT=0, FM=0}
      mask prompts: Constant "Value of Constant:"; FP "Fault Profile:"; FT "Fault Injection Time:"; FM "Fault Magnitude/Slope"
      mask TypeOptions FP: Nominal | Abrupt | Incipient
      mask Display: image(imread('Constant.png','BackgroundColor',[1 1 1]))
  MSe : Subsystem  mask{FP=Nominal, FT=0, FM=0}
      mask prompts: FP "Fault Profile:"; FT "Fault Injection Time:"; FM "Fault Magnitude/Slope/Bias/StuckAtValue"
      mask TypeOptions FP: Nominal | Abrupt | Incipient | Bias | StuckAt
      mask Display: image(imread('MSe.png','BackgroundColor',[1 1 1]))
  Mux : Mux  Inputs=3
  R : Subsystem  mask{R=1, FP=Nominal, FT=0, FM=0}
      mask prompts: R "Value of R:"; FP "Fault Profile:"; FT "Fault Injection Time:"; FM "Fault Magnitude/Slope"
      mask TypeOptions FP: Nominal | Abrupt | Incipient
      mask Display: image(imread('R.png','BackgroundColor',[1 1 1]))
  TrueValueMod : Subsystem
      mask Display: image(imread('fx.png','BackgroundColor',[1 1 1]))
  Value : Subsystem
      mask Display: image(imread('0.png','BackgroundColor',[1 1 1]))
  SensedValue : Outport
  wires:
  MSe/1(E) -> Value/1(u(1))
  Value/1(y(1)) -> MSe/1(F)
  Value/2(y(2)) -> R/1(In)
  R/1(Out) -> Value/2(u(2))
  TrueValueMod/1(y(1)) -> MSe/2(Se)
  Value/3(O) -> SensedValue/1
  Mux/1 -> TrueValueMod/1(u(1))
  TrueValue/1 -> Mux/1
  Gain/1(C) -> Mux/2
  Bias/1(C) -> Mux/3

== system VirtualADAPT/VirtualADAPTv1/PowerDistribution/ACLoadBank1/L1C/FAN3 ==
  EPIn0 : Inport
  OneJunction : Subsystem
      mask Display: image(imread('1.png','BackgroundColor',[1 1 1]))
  R : Subsystem  mask{R=195, FP=Nominal, FT=0, FM=0}
      mask prompts: R "Value of R:"; FP "Fault Profile:"; FT "Fault Injection Time:"; FM "Fault Magnitude/Slope"
      mask TypeOptions FP: Nominal | Abrupt | Incipient
      mask Display: image(imread('R.png','BackgroundColor',[1 1 1]))
  ZeroJunction : Subsystem
      mask Display: image(imread('0.png','BackgroundColor',[1 1 1]))
  EPOut0 : Outport
  Current : Outport  Port=2
  Voltage : Outport  Port=3
  wires:
  OneJunction/1(y(1)) -> ZeroJunction/1(u(1))
  ZeroJunction/1(y(1)) -> OneJunction/1(u(1))
  ZeroJunction/2(y(2)) -> R/1(In)
  R/1(Out) -> ZeroJunction/2(u(2))
  EPIn0/1 -> OneJunction/2(u(2))
  OneJunction/2(y(2)) -> EPOut0/1
  OneJunction/3(O) -> Current/1
  ZeroJunction/3(O) -> Voltage/1

== system VirtualADAPT/VirtualADAPTv1/PowerDistribution/ACLoadBank1/L1D/LGT8 ==
  EPIn0 : Inport
  CurrentJunc : Subsystem
      mask Display: image(imread('1.png','BackgroundColor',[1 1 1]))
  R : Subsystem  mask{R=251.19, FP=Nominal, FT=0, FM=0}
      mask prompts: R "Value of R:"; FP "Fault Profile:"; FT "Fault Injection Time:"; FM "Fault Magnitude/Slope"
      mask TypeOptions FP: Nominal | Abrupt | Incipient
      mask Display: image(imread('R.png','BackgroundColor',[1 1 1]))
  VoltageJunc : Subsystem
      mask Display: image(imread('0.png','BackgroundColor',[1 1 1]))
  EPOut0 : Outport
  Current : Outport  Port=2
  Voltage : Outport  Port=3
  wires:
  VoltageJunc/1(y(1)) -> R/1(In)
  R/1(Out) -> VoltageJunc/1(u(1))
  CurrentJunc/1(y(1)) -> VoltageJunc/2(u(2))
  VoltageJunc/2(y(2)) -> CurrentJunc/1(u(1))
  EPIn0/1 -> CurrentJunc/2(u(2))
  CurrentJunc/2(y(2)) -> EPOut0/1
  CurrentJunc/3(O) -> Current/1
  VoltageJunc/3(O) -> Voltage/1

== system VirtualADAPT/VirtualADAPTv1/PowerDistribution/ACLoadBank1/L1E/PMP2 ==
  InputIn0 : Inport
  GY : Subsystem  mask{GY=0.1419, FP=Nominal, FT=0, FM=0}
      mask prompts: GY "Value of GY:"; FP "Fault Profile:"; FT "Fault Injection Time:"; FM "Fault Magnitude/Slope"
      mask TypeOptions FP: Nominal | Abrupt | Incipient
      mask Display: image(imread('GY.png','BackgroundColor',[1 1 1]))
  Inductance : Subsystem  mask{I=6.9585, IC=0, FP=Nominal, FT=0, FM=0}
      mask prompts: I "Value of I:"; IC "Initial Condition:"; FP "Fault Profile:"; FT "Fault Injection Time:"; FM "Fault Magnitude/Slope"
      mask TypeOptions FP: Nominal | Abrupt | Incipient
      mask Display: image(imread('I.png','BackgroundColor',[1 1 1]))
  Inertia : Subsystem  mask{I=0.00068682, IC=0, FP=Nominal, FT=0, FM=0}
      mask prompts: I "Value of I:"; IC "Initial Condition:"; FP "Fault Profile:"; FT "Fault Injection Time:"; FM "Fault Magnitude/Slope"
      mask TypeOptions FP: Nominal | Abrupt | Incipient
      mask Display: image(imread('I.png','BackgroundColor',[1 1 1]))
  MotorCurrent : Subsystem
      mask Display: image(imread('1.png','BackgroundColor',[1 1 1]))
  PumpFlow : Subsystem
      mask Display: image(imread('1.png','BackgroundColor',[1 1 1]))
  PumpResistance : Subsystem  mask{R=0.0011115, FP=Nominal, FT=0, FM=0}
      mask prompts: R "Value of R:"; FP "Fault Profile:"; FT "Fault Injection Time:"; FM "Fault Magnitude/Slope"
      mask TypeOptions FP: Nominal | Abrupt | Incipient
      mask Display: image(imread('R.png','BackgroundColor',[1 1 1]))
  Resistance : Subsystem  mask{R=238.269, FP=Nominal, FT=0, FM=0}
      mask prompts: R "Value of R:"; FP "Fault Profile:"; FT "Fault Injection Time:"; FM "Fault Magnitude/Slope"
      mask TypeOptions FP: Nominal | Abrupt | Incipient
      mask Display: image(imread('R.png','BackgroundColor',[1 1 1]))
  InputOut0 : Outport
  Current : Outport  Port=2
  Flow : Outport  Port=3
  wires:
  MotorCurrent/1(y(1)) -> Inductance/1(E)
  Inductance/1(F) -> MotorCurrent/1(u(1))
  MotorCurrent/2(y(2)) -> GY/1(I1)
  GY/1(O1) -> MotorCurrent/2(u(2))
  PumpFlow/1(y(1)) -> Inertia/1(E)
  Inertia/1(F) -> PumpFlow/1(u(1))
  PumpFlow/2(y(2)) -> PumpResistance/1(In)
  PumpResistance/1(Out) -> PumpFlow/2(u(2))
  MotorCurrent/3(y(3)) -> Resistance/1(In)
  Resistance/1(Out) -> MotorCurrent/3(u(3))
  GY/2(O2) -> PumpFlow/3(u(3))
  PumpFlow/3(y(3)) -> GY/2(I2)
  InputIn0/1 -> MotorCurrent/4(u(4))
  MotorCurrent/4(y(4)) -> InputOut0/1
  MotorCurrent/5(O) -> Current/1
  PumpFlow/4(O) -> Flow/1

== system VirtualADAPT/VirtualADAPTv1/PowerDistribution/ACLoadBank1/L1E/SensorFT525 ==
  TrueValue : Inport
  Bias : Subsystem  mask{Constant=-0.10163, FP=Nominal, FT=0, FM=0}
      mask prompts: Constant "Value of Constant:"; FP "Fault Profile:"; FT "Fault Injection Time:"; FM "Fault Magnitude/Slope"
      mask TypeOptions FP: Nominal | Abrupt | Incipient
      mask Display: image(imread('Constant.png','BackgroundColor',[1 1 1]))
  Gain : Subsystem  mask{Constant=3.195, FP=Nominal, FT=0, FM=0}
      mask prompts: Constant "Value of Constant:"; FP "Fault Profile:"; FT "Fault Injection Time:"; FM "Fault Magnitude/Slope"
      mask TypeOptions FP: Nominal | Abrupt | Incipient
      mask Display: image(imread('Constant.png','BackgroundColor',[1 1 1]))
  MSe : Subsystem  mask{FP=Nominal, FT=0, FM=0}
      mask prompts: FP "Fault Profile:"; FT "Fault Injection Time:"; FM "Fault Magnitude/Slope/Bias/StuckAtValue"
      mask TypeOptions FP: Nominal | Abrupt | Incipient | Bias | StuckAt
      mask Display: image(imread('MSe.png','BackgroundColor',[1 1 1]))
  Mux : Mux  Inputs=3
  R : Subsystem  mask{R=1, FP=Nominal, FT=0, FM=0}
      mask prompts: R "Value of R:"; FP "Fault Profile:"; FT "Fault Injection Time:"; FM "Fault Magnitude/Slope"
      mask TypeOptions FP: Nominal | Abrupt | Incipient
      mask Display: image(imread('R.png','BackgroundColor',[1 1 1]))
  TrueValueMod : Subsystem
      mask Display: image(imread('fx.png','BackgroundColor',[1 1 1]))
  Value : Subsystem
      mask Display: image(imread('0.png','BackgroundColor',[1 1 1]))
  SensedValue : Outport
  wires:
  MSe/1(E) -> Value/1(u(1))
  Value/1(y(1)) -> MSe/1(F)
  Value/2(y(2)) -> R/1(In)
  R/1(Out) -> Value/2(u(2))
  TrueValueMod/1(y(1)) -> MSe/2(Se)
  Value/3(O) -> SensedValue/1
  Gain/1(C) -> Mux/2
  Bias/1(C) -> Mux/3
  Mux/1 -> TrueValueMod/1(u(1))
  TrueValue/1 -> Mux/1

== system VirtualADAPT/VirtualADAPTv1/PowerDistribution/ACLoadBank1/L1F/LGT4 ==
  EPIn0 : Inport
  AmbientTemp : Inport  Port=2
  ComputePower : Subsystem
      mask Display: image(imread('fx.png','BackgroundColor',[1 1 1]))
  I : Subsystem
      mask Display: image(imread('1.png','BackgroundColor',[1 1 1]))
  R : Subsystem  mask{R=257.71, FP=Nominal, FT=0, FM=0}
      mask prompts: R "Value of R:"; FP "Fault Profile:"; FT "Fault Injection Time:"; FM "Fault Magnitude/Slope"
      mask TypeOptions FP: Nominal | Abrupt | Incipient
      mask Display: image(imread('R.png','BackgroundColor',[1 1 1]))
  TemperatureModel : Subsystem
  V : Subsystem
      mask Display: image(imread('0.png','BackgroundColor',[1 1 1]))
  EPOut0 : Outport
  Power : Outport  Port=2
  Current : Outport  Port=3
  Temperature : Outport  Port=4
  wires:
  V/1(y(1)) -> R/1(In)
  R/1(Out) -> V/1(u(1))
  I/1(y(1)) -> V/2(u(2))
  V/2(y(2)) -> I/1(u(1))
  EPIn0/1 -> I/2(u(2))
  I/2(y(2)) -> EPOut0/1
  I/3(O) -> ComputePower/1(u(1)), Current/1
  V/3(O) -> ComputePower/2(u(2))
  ComputePower/1(y(1)) -> TemperatureModel/1(Power), Power/1
  TemperatureModel/1(Temp) -> Temperature/1
  AmbientTemp/1 -> TemperatureModel/2(AmbientTemp)

== system VirtualADAPT/VirtualADAPTv1/PowerDistribution/ACLoadBank1/L1F/SensorTE511 ==
  TrueValue : Inport
  MSe : Subsystem  mask{FP=Nominal, FT=0, FM=0}
      mask prompts: FP "Fault Profile:"; FT "Fault Injection Time:"; FM "Fault Magnitude/Slope/Bias/StuckAtValue"
      mask TypeOptions FP: Nominal | Abrupt | Incipient | Bias | StuckAt
      mask Display: image(imread('MSe.png','BackgroundColor',[1 1 1]))
  R : Subsystem  mask{R=1, FP=Nominal, FT=0, FM=0}
      mask prompts: R "Value of R:"; FP "Fault Profile:"; FT "Fault Injection Time:"; FM "Fault Magnitude/Slope"
      mask TypeOptions FP: Nominal | Abrupt | Incipient
      mask Display: image(imread('R.png','BackgroundColor',[1 1 1]))
  TempBias : Subsystem  mask{Constant=-2.33, FP=Nominal, FT=0, FM=0}
      mask prompts: Constant "Value of Constant:"; FP "Fault Profile:"; FT "Fault Injection Time:"; FM "Fault Magnitude/Slope"
      mask TypeOptions FP: Nominal | Abrupt | Incipient
      mask Display: image(imread('Constant.png','BackgroundColor',[1 1 1]))
  TrueValueMod : Subsystem
      mask Display: image(imread('fx.png','BackgroundColor',[1 1 1]))
  Value : Subsystem
      mask Display: image(imread('0.png','BackgroundColor',[1 1 1]))
  SensedValue : Outport
  wires:
  MSe/1(E) -> Value/1(u(1))
  Value/1(y(1)) -> MSe/1(F)
  Value/2(y(2)) -> R/1(In)
  R/1(Out) -> Value/2(u(2))
  TrueValueMod/1(y(1)) -> MSe/2(Se)
  TempBias/1(C) -> TrueValueMod/1(u(1))
  TrueValue/1 -> TrueValueMod/2(u(2))
  Value/3(O) -> SensedValue/1

== system VirtualADAPT/VirtualADAPTv1/PowerDistribution/ACLoadBank1/RelayEY170/ParallelConn ==
  u(1) : Inport
  u(2) : Inport  Port=2
  u(3) : Inport  Port=3
  Adder : Sum  Inputs=-  SaturateOnIntegerOverflow=on  InputSameDT=on  OutDataTypeStr=Inherit: Same as first input
  Gain : Gain  Gain=-1  SaturateOnIntegerOverflow=on  OutDataTypeStr=Inherit: Same as input  ParamDataTypeStr=Inherit: Same as input
  Mux : Mux  Inputs=3
  OffGuard : Fcn  Expr=(!u(3))
  OnGuard : Fcn  Expr=(u(3))
  Product1 : Product  SaturateOnIntegerOverflow=on  RndMeth=Zero  InputSameDT=on  OutDataTypeStr=Inherit: Same as first input
  Product2 : Product  SaturateOnIntegerOverflow=on  RndMeth=Zero  InputSameDT=on  OutDataTypeStr=Inherit: Same as first input
  determineState : Subsystem
  y(1) : Outport
  y(2) : Outport  Port=2
  O : Outport  Port=3
  State : Outport  Port=4
  wires:
  Adder/1 -> Gain/1
  u(3)/1 -> Mux/3
  Mux/1 -> OnGuard/1, OffGuard/1
  OnGuard/1 -> determineState/1(In1)
  OffGuard/1 -> determineState/2(In2)
  u(1)/1 -> Product1/1
  determineState/1(Out1) -> Product1/2, Product2/2, State/1
  Product1/1 -> O/1, y(2)/1
  Gain/1 -> Product2/1
  Product2/1 -> y(1)/1
  u(2)/1 -> Adder/1

== system VirtualADAPT/VirtualADAPTv1/PowerDistribution/ACLoadBank1/RelayEY170/Relay ==
  u(1) : Inport
  u(2) : Inport  Port=2
  u(3) : Inport  Port=3
  Adder : Sum  Inputs=+  SaturateOnIntegerOverflow=on  InputSameDT=on  OutDataTypeStr=Inherit: Same as first input
  Gain : Gain  SaturateOnIntegerOverflow=on  OutDataTypeStr=Inherit: Same as input  ParamDataTypeStr=Inherit: Same as input
  Mux : Mux  Inputs=3
  OffGuard : Fcn  Expr=(!u(3))
  OnGuard : Fcn  Expr=(u(3))
  Product1 : Product  SaturateOnIntegerOverflow=on  RndMeth=Zero  InputSameDT=on  OutDataTypeStr=Inherit: Same as first input
  Product2 : Product  SaturateOnIntegerOverflow=on  RndMeth=Zero  InputSameDT=on  OutDataTypeStr=Inherit: Same as first input
  determineState : Subsystem
  y(1) : Outport
  y(2) : Outport  Port=2
  O : Outport  Port=3
  State : Outport  Port=4
  wires:
  Adder/1 -> Gain/1
  u(3)/1 -> Mux/3
  Mux/1 -> OnGuard/1, OffGuard/1
  OnGuard/1 -> determineState/1(In1)
  OffGuard/1 -> determineState/2(In2)
  u(1)/1 -> Product1/1
  determineState/1(Out1) -> Product1/2, Product2/2, State/1
  Product1/1 -> O/1, y(2)/1
  Gain/1 -> Product2/1
  Product2/1 -> y(1)/1
  u(2)/1 -> Adder/1

== system VirtualADAPT/VirtualADAPTv1/PowerDistribution/ACLoadBank1/RelayEY170/Sensor ==
  TrueValue : Inport
  MSe : Subsystem  mask{FP=Nominal, FT=0, FM=0}
      mask prompts: FP "Fault Profile:"; FT "Fault Injection Time:"; FM "Fault Magnitude/Slope/Bias/StuckAtValue"
      mask TypeOptions FP: Nominal | Abrupt | Incipient | Bias | StuckAt
      mask Display: image(imread('MSe.png','BackgroundColor',[1 1 1]))
  R : Subsystem  mask{R=1, FP=Nominal, FT=0, FM=0}
      mask prompts: R "Value of R:"; FP "Fault Profile:"; FT "Fault Injection Time:"; FM "Fault Magnitude/Slope"
      mask TypeOptions FP: Nominal | Abrupt | Incipient
      mask Display: image(imread('R.png','BackgroundColor',[1 1 1]))
  TrueValueMod : Subsystem
      mask Display: image(imread('fx.png','BackgroundColor',[1 1 1]))
  Value : Subsystem
      mask Display: image(imread('0.png','BackgroundColor',[1 1 1]))
  SensedValue : Outport
  wires:
  Value/1(y(1)) -> R/1(In)
  R/1(Out) -> Value/1(u(1))
  MSe/1(E) -> Value/2(u(2))
  Value/2(y(2)) -> MSe/1(F)
  TrueValueMod/1(y(1)) -> MSe/2(Se)
  TrueValue/1 -> TrueValueMod/1(u(1))
  Value/3(O) -> SensedValue/1

== system VirtualADAPT/VirtualADAPTv1/PowerDistribution/ACLoadBank1/RelayEY171/ParallelConn ==
  u(1) : Inport
  u(2) : Inport  Port=2
  u(3) : Inport  Port=3
  Adder : Sum  Inputs=-  SaturateOnIntegerOverflow=on  InputSameDT=on  OutDataTypeStr=Inherit: Same as first input
  Gain : Gain  Gain=-1  SaturateOnIntegerOverflow=on  OutDataTypeStr=Inherit: Same as input  ParamDataTypeStr=Inherit: Same as input
  Mux : Mux  Inputs=3
  OffGuard : Fcn  Expr=(!u(3))
  OnGuard : Fcn  Expr=(u(3))
  Product1 : Product  SaturateOnIntegerOverflow=on  RndMeth=Zero  InputSameDT=on  OutDataTypeStr=Inherit: Same as first input
  Product2 : Product  SaturateOnIntegerOverflow=on  RndMeth=Zero  InputSameDT=on  OutDataTypeStr=Inherit: Same as first input
  determineState : Subsystem
  y(1) : Outport
  y(2) : Outport  Port=2
  O : Outport  Port=3
  State : Outport  Port=4
  wires:
  Adder/1 -> Gain/1
  u(3)/1 -> Mux/3
  Mux/1 -> OnGuard/1, OffGuard/1
  OnGuard/1 -> determineState/1(In1)
  OffGuard/1 -> determineState/2(In2)
  u(1)/1 -> Product1/1
  determineState/1(Out1) -> Product1/2, Product2/2, State/1
  Product1/1 -> O/1, y(2)/1
  Gain/1 -> Product2/1
  Product2/1 -> y(1)/1
  u(2)/1 -> Adder/1

== system VirtualADAPT/VirtualADAPTv1/PowerDistribution/ACLoadBank1/RelayEY171/Relay ==
  u(1) : Inport
  u(2) : Inport  Port=2
  u(3) : Inport  Port=3
  Adder : Sum  Inputs=+  SaturateOnIntegerOverflow=on  InputSameDT=on  OutDataTypeStr=Inherit: Same as first input
  Gain : Gain  SaturateOnIntegerOverflow=on  OutDataTypeStr=Inherit: Same as input  ParamDataTypeStr=Inherit: Same as input
  Mux : Mux  Inputs=3
  OffGuard : Fcn  Expr=(!u(3))
  OnGuard : Fcn  Expr=(u(3))
  Product1 : Product  SaturateOnIntegerOverflow=on  RndMeth=Zero  InputSameDT=on  OutDataTypeStr=Inherit: Same as first input
  Product2 : Product  SaturateOnIntegerOverflow=on  RndMeth=Zero  InputSameDT=on  OutDataTypeStr=Inherit: Same as first input
  determineState : Subsystem
  y(1) : Outport
  y(2) : Outport  Port=2
  O : Outport  Port=3
  State : Outport  Port=4
  wires:
  Adder/1 -> Gain/1
  u(3)/1 -> Mux/3
  Mux/1 -> OnGuard/1, OffGuard/1
  OnGuard/1 -> determineState/1(In1)
  OffGuard/1 -> determineState/2(In2)
  u(1)/1 -> Product1/1
  determineState/1(Out1) -> Product1/2, Product2/2, State/1
  Product1/1 -> O/1, y(2)/1
  Gain/1 -> Product2/1
  Product2/1 -> y(1)/1
  u(2)/1 -> Adder/1

== system VirtualADAPT/VirtualADAPTv1/PowerDistribution/ACLoadBank1/RelayEY171/Sensor ==
  TrueValue : Inport
  MSe : Subsystem  mask{FP=Nominal, FT=0, FM=0}
      mask prompts: FP "Fault Profile:"; FT "Fault Injection Time:"; FM "Fault Magnitude/Slope/Bias/StuckAtValue"
      mask TypeOptions FP: Nominal | Abrupt | Incipient | Bias | StuckAt
      mask Display: image(imread('MSe.png','BackgroundColor',[1 1 1]))
  R : Subsystem  mask{R=1, FP=Nominal, FT=0, FM=0}
      mask prompts: R "Value of R:"; FP "Fault Profile:"; FT "Fault Injection Time:"; FM "Fault Magnitude/Slope"
      mask TypeOptions FP: Nominal | Abrupt | Incipient
      mask Display: image(imread('R.png','BackgroundColor',[1 1 1]))
  TrueValueMod : Subsystem
      mask Display: image(imread('fx.png','BackgroundColor',[1 1 1]))
  Value : Subsystem
      mask Display: image(imread('0.png','BackgroundColor',[1 1 1]))
  SensedValue : Outport
  wires:
  Value/1(y(1)) -> R/1(In)
  R/1(Out) -> Value/1(u(1))
  MSe/1(E) -> Value/2(u(2))
  Value/2(y(2)) -> MSe/1(F)
  TrueValueMod/1(y(1)) -> MSe/2(Se)
  TrueValue/1 -> TrueValueMod/1(u(1))
  Value/3(O) -> SensedValue/1

== system VirtualADAPT/VirtualADAPTv1/PowerDistribution/ACLoadBank1/RelayEY172/ParallelConn ==
  u(1) : Inport
  u(2) : Inport  Port=2
  u(3) : Inport  Port=3
  Adder : Sum  Inputs=-  SaturateOnIntegerOverflow=on  InputSameDT=on  OutDataTypeStr=Inherit: Same as first input
  Gain : Gain  Gain=-1  SaturateOnIntegerOverflow=on  OutDataTypeStr=Inherit: Same as input  ParamDataTypeStr=Inherit: Same as input
  Mux : Mux  Inputs=3
  OffGuard : Fcn  Expr=(!u(3))
  OnGuard : Fcn  Expr=(u(3))
  Product1 : Product  SaturateOnIntegerOverflow=on  RndMeth=Zero  InputSameDT=on  OutDataTypeStr=Inherit: Same as first input
  Product2 : Product  SaturateOnIntegerOverflow=on  RndMeth=Zero  InputSameDT=on  OutDataTypeStr=Inherit: Same as first input
  determineState : Subsystem
  y(1) : Outport
  y(2) : Outport  Port=2
  O : Outport  Port=3
  State : Outport  Port=4
  wires:
  Adder/1 -> Gain/1
  u(3)/1 -> Mux/3
  Mux/1 -> OnGuard/1, OffGuard/1
  OnGuard/1 -> determineState/1(In1)
  OffGuard/1 -> determineState/2(In2)
  u(1)/1 -> Product1/1
  determineState/1(Out1) -> Product1/2, Product2/2, State/1
  Product1/1 -> O/1, y(2)/1
  Gain/1 -> Product2/1
  Product2/1 -> y(1)/1
  u(2)/1 -> Adder/1

== system VirtualADAPT/VirtualADAPTv1/PowerDistribution/ACLoadBank1/RelayEY172/Relay ==
  u(1) : Inport
  u(2) : Inport  Port=2
  u(3) : Inport  Port=3
  Adder : Sum  Inputs=+  SaturateOnIntegerOverflow=on  InputSameDT=on  OutDataTypeStr=Inherit: Same as first input
  Gain : Gain  SaturateOnIntegerOverflow=on  OutDataTypeStr=Inherit: Same as input  ParamDataTypeStr=Inherit: Same as input
  Mux : Mux  Inputs=3
  OffGuard : Fcn  Expr=(!u(3))
  OnGuard : Fcn  Expr=(u(3))
  Product1 : Product  SaturateOnIntegerOverflow=on  RndMeth=Zero  InputSameDT=on  OutDataTypeStr=Inherit: Same as first input
  Product2 : Product  SaturateOnIntegerOverflow=on  RndMeth=Zero  InputSameDT=on  OutDataTypeStr=Inherit: Same as first input
  determineState : Subsystem
  y(1) : Outport
  y(2) : Outport  Port=2
  O : Outport  Port=3
  State : Outport  Port=4
  wires:
  Adder/1 -> Gain/1
  u(3)/1 -> Mux/3
  Mux/1 -> OnGuard/1, OffGuard/1
  OnGuard/1 -> determineState/1(In1)
  OffGuard/1 -> determineState/2(In2)
  u(1)/1 -> Product1/1
  determineState/1(Out1) -> Product1/2, Product2/2, State/1
  Product1/1 -> O/1, y(2)/1
  Gain/1 -> Product2/1
  Product2/1 -> y(1)/1
  u(2)/1 -> Adder/1

== system VirtualADAPT/VirtualADAPTv1/PowerDistribution/ACLoadBank1/RelayEY172/Sensor ==
  TrueValue : Inport
  MSe : Subsystem  mask{FP=Nominal, FT=0, FM=0}
      mask prompts: FP "Fault Profile:"; FT "Fault Injection Time:"; FM "Fault Magnitude/Slope/Bias/StuckAtValue"
      mask TypeOptions FP: Nominal | Abrupt | Incipient | Bias | StuckAt
      mask Display: image(imread('MSe.png','BackgroundColor',[1 1 1]))
  R : Subsystem  mask{R=1, FP=Nominal, FT=0, FM=0}
      mask prompts: R "Value of R:"; FP "Fault Profile:"; FT "Fault Injection Time:"; FM "Fault Magnitude/Slope"
      mask TypeOptions FP: Nominal | Abrupt | Incipient
      mask Display: image(imread('R.png','BackgroundColor',[1 1 1]))
  TrueValueMod : Subsystem
      mask Display: image(imread('fx.png','BackgroundColor',[1 1 1]))
  Value : Subsystem
      mask Display: image(imread('0.png','BackgroundColor',[1 1 1]))
  SensedValue : Outport
  wires:
  Value/1(y(1)) -> R/1(In)
  R/1(Out) -> Value/1(u(1))
  MSe/1(E) -> Value/2(u(2))
  Value/2(y(2)) -> MSe/1(F)
  TrueValueMod/1(y(1)) -> MSe/2(Se)
  TrueValue/1 -> TrueValueMod/1(u(1))
  Value/3(O) -> SensedValue/1
